simulink model "gmrt_002_muxdelay"  (Simulink 7.5) (continued)
== system gmrt_002_muxdelay == (continued)
      mask init:
      | pfb_fir_mux_init(gcb, ...
      |     'PFBSize', PFBSize, ...
      |     'mux_inputs', mux_inputs, ...
      |     'TotalTaps', TotalTaps, ...
      |     'WindowType', WindowType, ...
      |     'n_inputs', n_inputs, ...
      |     'MakeBiplex', MakeBiplex, ...
      |     'BitWidthIn', BitWidthIn, ...
      |     'BitWidthOut', BitWidthOut, ...
      |     'CoeffBitWidth', CoeffBitWidth, ...
      |     'CoeffDistMem', CoeffDistMem, ...
      |     'add_latency', add_latency, ...
      |     'mult_latency', mult_latency, ...
      |     'bram_latency', bram_latency, ...
      |     'quantization', quantization, ...
      |     'fwidth', fwidth,...
      |     'specify_mult', specify_mult,...
      |     'mult_spec', mult_spec);
      settings: AttributesFormatString=taps=4, add_latency=1
      mask Help: eval('xlWeb(''http://casper.berkeley.edu/wiki/Pfb_fir'')')
      mask StyleString: edit,edit,edit,popup(bartlett|barthannwin|blackman|blackmanharris|bohamwin|chebwin|flattopwin|gausswin|hamming|hann|kaiser|nuttallwin|parzenwin|rectwin|tukeywin|triang|userwindow),edit,edit,edit,edit,edit,edit,edit,edit,edit,popup(Truncate|Round  (unbiased: +/- Inf)|Round  (unbiased: Even Values)),edit,checkbox,edit
      mask CallbackString:
      | ||%num_taps = eval(get_param(gcb, 'TotalTaps'));
      | %old_vec = eval(get_param(gcb, 'mult_spec'));
      | 
      | %vec = 2.*ones(1,num_taps);
      | %old_size = length(old_vec);
      | 
      | %if(old_size <= num_taps),
      | %   new_size = old_size;
      | %else,
      | %    new_size = num_taps;
      | %end
      | 
      | %for n = 1:new_size, vec(n) = old_vec(n); end
      | %set_param(gcb, 'mult_spec', mat2str(vec));||||||||||||%num_taps = eval(get_param(gcb, 'TotalTaps'));
      | %old_vec = eval(get_param(gcb, 'mult_spec'));
      | 
      | %vec = 2.*ones(1,num_taps);
      | %old_size = length(old_vec);
      | 
      | %if(old_size <= num_taps),
      | %    new_size = old_size;
      | %else,
      | %    new_size = num_taps;
      | %end
      | 
      | %for n = 1:new_size, vec(n) = old_vec(n); end
      | %set_param(gcb, 'mult_spec', mat2str(vec));|%specify_mult = get_param(gcb,'specify_mult');
      | %if( strcmp(specify_mult,'off')),
      | %    set_param(gcb, 'MaskEnables', {'on','on','on','on','on','on','on','on','on','on','on','on','on','on','on','off'});
      | %else
      | %    set_param(gcb, 'MaskEnables', {'on','on','on','on','on','on','on','on','on','on','on','on','on','on','on','on'});
      | %end
      | 
      | |%specify_mult = get_param(gcb,'specify_mult');
      | %if( strcmp(specify_mult,'off')),
      | %    set_param(gcb, 'MaskEnables', {'on','on','on','on','on','on','on','on','on','on','on','on','on','on','on','off'});
      | %else
      | %    set_param(gcb, 'MaskEnables', {'on','on','on','on','on','on','on','on','on','on','on','on','on','on','on','on'});
      | %end
  pfb_fir_mux2 : Subsystem  AncestorBlock=casper_library_pfbs/pfb_fir_mux  mask(pfb_fir_mux){PFBSize=10, mux_inputs=2, TotalTaps=4, WindowType=hamming, n_inputs=0, MakeBiplex=1, BitWidthIn=12, BitWidthOut=18, CoeffBitWidth=18, CoeffDistMem=0, add_latency=1, mult_latency=2, bram_latency=3, quantization=Round  (unbiased: +/- Inf), fwidth=1, specify_mult=off, mult_spec=[2                                      2]}
      mask prompts: PFBSize "Size of PFB: (2^? pnts)"; mux_inputs "Signals per input (2^?)"; TotalTaps "Total Number of Taps:"; WindowType "Windowing Function:"; n_inputs "Number of Simultaneous Inputs: (2^?)"; MakeBiplex "Make Biplex"; BitWidthIn "Input Bitwidth:"; BitWidthOut "Output Bitwidth:"; CoeffBitWidth "Coefficient Bitwidth:"; CoeffDistMem "Use Distributed Memory for Coeffs"; add_latency "Add Latency"; mult_latency "Mult Latency"; bram_latency "BRAM Latency"; quantization "Quantization Behavior"; fwidth "Bin Width Scaling (normal=1)"; specify_mult "Specify multiplier use (behavioral if not specified)"; mult_spec "Multiplier specification (0=core, 1=embedded, 2=behavioural) (left=1st tap)"
      mask init: same as gmrt_002_muxdelay/pfb_fir_mux1
      settings: AttributesFormatString=taps=4, add_latency=1
      mask Help: eval('xlWeb(''http://casper.berkeley.edu/wiki/Pfb_fir'')')
      mask StyleString: edit,edit,edit,popup(bartlett|barthannwin|blackman|blackmanharris|bohamwin|chebwin|flattopwin|gausswin|hamming|hann|kaiser|nuttallwin|parzenwin|rectwin|tukeywin|triang|userwindow),edit,edit,edit,edit,edit,edit,edit,edit,edit,popup(Truncate|Round  (unbiased: +/- Inf)|Round  (unbiased: Even Values)),edit,checkbox,edit
      mask CallbackString:
      | ||%num_taps = eval(get_param(gcb, 'TotalTaps'));
      | %old_vec = eval(get_param(gcb, 'mult_spec'));
      | 
      | %vec = 2.*ones(1,num_taps);
      | %old_size = length(old_vec);
      | 
      | %if(old_size <= num_taps),
      | %   new_size = old_size;
      | %else,
      | %    new_size = num_taps;
      | %end
      | 
      | %for n = 1:new_size, vec(n) = old_vec(n); end
      | %set_param(gcb, 'mult_spec', mat2str(vec));||||||||||||%num_taps = eval(get_param(gcb, 'TotalTaps'));
      | %old_vec = eval(get_param(gcb, 'mult_spec'));
      | 
      | %vec = 2.*ones(1,num_taps);
      | %old_size = length(old_vec);
      | 
      | %if(old_size <= num_taps),
      | %    new_size = old_size;
      | %else,
      | %    new_size = num_taps;
      | %end
      | 
      | %for n = 1:new_size, vec(n) = old_vec(n); end
      | %set_param(gcb, 'mult_spec', mat2str(vec));|%specify_mult = get_param(gcb,'specify_mult');
      | %if( strcmp(specify_mult,'off')),
      | %    set_param(gcb, 'MaskEnables', {'on','on','on','on','on','on','on','on','on','on','on','on','on','on','on','off'});
      | %else
      | %    set_param(gcb, 'MaskEnables', {'on','on','on','on','on','on','on','on','on','on','on','on','on','on','on','on'});
      | %end
      | 
      | |%specify_mult = get_param(gcb,'specify_mult');
      | %if( strcmp(specify_mult,'off')),
      | %    set_param(gcb, 'MaskEnables', {'on','on','on','on','on','on','on','on','on','on','on','on','on','on','on','off'});
      | %else
      | %    set_param(gcb, 'MaskEnables', {'on','on','on','on','on','on','on','on','on','on','on','on','on','on','on','on'});
      | %end
  pfb_fir_mux3 : Subsystem  AncestorBlock=casper_library_pfbs/pfb_fir_mux  mask(pfb_fir_mux){PFBSize=10, mux_inputs=2, TotalTaps=4, WindowType=hamming, n_inputs=0, MakeBiplex=1, BitWidthIn=12, BitWidthOut=18, CoeffBitWidth=18, CoeffDistMem=0, add_latency=1, mult_latency=2, bram_latency=3, quantization=Round  (unbiased: +/- Inf), fwidth=1, specify_mult=off, mult_spec=[2                                      2]}
      mask prompts: PFBSize "Size of PFB: (2^? pnts)"; mux_inputs "Signals per input (2^?)"; TotalTaps "Total Number of Taps:"; WindowType "Windowing Function:"; n_inputs "Number of Simultaneous Inputs: (2^?)"; MakeBiplex "Make Biplex"; BitWidthIn "Input Bitwidth:"; BitWidthOut "Output Bitwidth:"; CoeffBitWidth "Coefficient Bitwidth:"; CoeffDistMem "Use Distributed Memory for Coeffs"; add_latency "Add Latency"; mult_latency "Mult Latency"; bram_latency "BRAM Latency"; quantization "Quantization Behavior"; fwidth "Bin Width Scaling (normal=1)"; specify_mult "Specify multiplier use (behavioral if not specified)"; mult_spec "Multiplier specification (0=core, 1=embedded, 2=behavioural) (left=1st tap)"
      mask init: same as gmrt_002_muxdelay/pfb_fir_mux1
      settings: AttributesFormatString=taps=4, add_latency=1
      mask Help: eval('xlWeb(''http://casper.berkeley.edu/wiki/Pfb_fir'')')
      mask StyleString: edit,edit,edit,popup(bartlett|barthannwin|blackman|blackmanharris|bohamwin|chebwin|flattopwin|gausswin|hamming|hann|kaiser|nuttallwin|parzenwin|rectwin|tukeywin|triang|userwindow),edit,edit,edit,edit,edit,edit,edit,edit,edit,popup(Truncate|Round  (unbiased: +/- Inf)|Round  (unbiased: Even Values)),edit,checkbox,edit
      mask CallbackString:
      | ||%num_taps = eval(get_param(gcb, 'TotalTaps'));
      | %old_vec = eval(get_param(gcb, 'mult_spec'));
      | 
      | %vec = 2.*ones(1,num_taps);
      | %old_size = length(old_vec);
      | 
      | %if(old_size <= num_taps),
      | %   new_size = old_size;
      | %else,
      | %    new_size = num_taps;
      | %end
      | 
      | %for n = 1:new_size, vec(n) = old_vec(n); end
      | %set_param(gcb, 'mult_spec', mat2str(vec));||||||||||||%num_taps = eval(get_param(gcb, 'TotalTaps'));
      | %old_vec = eval(get_param(gcb, 'mult_spec'));
      | 
      | %vec = 2.*ones(1,num_taps);
      | %old_size = length(old_vec);
      | 
      | %if(old_size <= num_taps),
      | %    new_size = old_size;
      | %else,
      | %    new_size = num_taps;
      | %end
      | 
      | %for n = 1:new_size, vec(n) = old_vec(n); end
      | %set_param(gcb, 'mult_spec', mat2str(vec));|%specify_mult = get_param(gcb,'specify_mult');
      | %if( strcmp(specify_mult,'off')),
      | %    set_param(gcb, 'MaskEnables', {'on','on','on','on','on','on','on','on','on','on','on','on','on','on','on','off'});
      | %else
      | %    set_param(gcb, 'MaskEnables', {'on','on','on','on','on','on','on','on','on','on','on','on','on','on','on','on'});
      | %end
      | 
      | |%specify_mult = get_param(gcb,'specify_mult');
      | %if( strcmp(specify_mult,'off')),
      | %    set_param(gcb, 'MaskEnables', {'on','on','on','on','on','on','on','on','on','on','on','on','on','on','on','off'});
      | %else
      | %    set_param(gcb, 'MaskEnables', {'on','on','on','on','on','on','on','on','on','on','on','on','on','on','on','on'});
      | %end
  pfb_fir_mux4 : Subsystem  AncestorBlock=casper_library_pfbs/pfb_fir_mux  mask(pfb_fir_mux){PFBSize=10, mux_inputs=2, TotalTaps=4, WindowType=hamming, n_inputs=0, MakeBiplex=1, BitWidthIn=12, BitWidthOut=18, CoeffBitWidth=18, CoeffDistMem=0, add_latency=1, mult_latency=2, bram_latency=3, quantization=Round  (unbiased: +/- Inf), fwidth=1, specify_mult=off, mult_spec=[2                                      2]}
      mask prompts: PFBSize "Size of PFB: (2^? pnts)"; mux_inputs "Signals per input (2^?)"; TotalTaps "Total Number of Taps:"; WindowType "Windowing Function:"; n_inputs "Number of Simultaneous Inputs: (2^?)"; MakeBiplex "Make Biplex"; BitWidthIn "Input Bitwidth:"; BitWidthOut "Output Bitwidth:"; CoeffBitWidth "Coefficient Bitwidth:"; CoeffDistMem "Use Distributed Memory for Coeffs"; add_latency "Add Latency"; mult_latency "Mult Latency"; bram_latency "BRAM Latency"; quantization "Quantization Behavior"; fwidth "Bin Width Scaling (normal=1)"; specify_mult "Specify multiplier use (behavioral if not specified)"; mult_spec "Multiplier specification (0=core, 1=embedded, 2=behavioural) (left=1st tap)"
      mask init: same as gmrt_002_muxdelay/pfb_fir_mux1
      settings: AttributesFormatString=taps=4, add_latency=1
      mask Help: eval('xlWeb(''http://casper.berkeley.edu/wiki/Pfb_fir'')')
      mask StyleString: edit,edit,edit,popup(bartlett|barthannwin|blackman|blackmanharris|bohamwin|chebwin|flattopwin|gausswin|hamming|hann|kaiser|nuttallwin|parzenwin|rectwin|tukeywin|triang|userwindow),edit,edit,edit,edit,edit,edit,edit,edit,edit,popup(Truncate|Round  (unbiased: +/- Inf)|Round  (unbiased: Even Values)),edit,checkbox,edit
      mask CallbackString:
      | ||%num_taps = eval(get_param(gcb, 'TotalTaps'));
      | %old_vec = eval(get_param(gcb, 'mult_spec'));
      | 
      | %vec = 2.*ones(1,num_taps);
      | %old_size = length(old_vec);
      | 
      | %if(old_size <= num_taps),
      | %   new_size = old_size;
      | %else,
      | %    new_size = num_taps;
      | %end
      | 
      | %for n = 1:new_size, vec(n) = old_vec(n); end
      | %set_param(gcb, 'mult_spec', mat2str(vec));||||||||||||%num_taps = eval(get_param(gcb, 'TotalTaps'));
      | %old_vec = eval(get_param(gcb, 'mult_spec'));
      | 
      | %vec = 2.*ones(1,num_taps);
      | %old_size = length(old_vec);
      | 
      | %if(old_size <= num_taps),
      | %    new_size = old_size;
      | %else,
      | %    new_size = num_taps;
      | %end
      | 
      | %for n = 1:new_size, vec(n) = old_vec(n); end
      | %set_param(gcb, 'mult_spec', mat2str(vec));|%specify_mult = get_param(gcb,'specify_mult');
      | %if( strcmp(specify_mult,'off')),
      | %    set_param(gcb, 'MaskEnables', {'on','on','on','on','on','on','on','on','on','on','on','on','on','on','on','off'});
      | %else
      | %    set_param(gcb, 'MaskEnables', {'on','on','on','on','on','on','on','on','on','on','on','on','on','on','on','on'});
      | %end
      | 
      | |%specify_mult = get_param(gcb,'specify_mult');
      | %if( strcmp(specify_mult,'off')),
      | %    set_param(gcb, 'MaskEnables', {'on','on','on','on','on','on','on','on','on','on','on','on','on','on','on','off'});
      | %else
      | %    set_param(gcb, 'MaskEnables', {'on','on','on','on','on','on','on','on','on','on','on','on','on','on','on','on'});
      | %end
  phase_EQ1 : Subsystem  mask{N=10+5 - 2, bram_lat=2, mult_lat=3, add_lat=2, chans_per_coeff=2}
      mask prompts: N "number of coefficients (2^?)"; bram_lat "bram latency"; mult_lat "multiplier latency"; add_lat "adder latency"; chans_per_coeff "channels per coeff (2^?)"
  pipeline : Subsystem  AncestorBlock=casper_library/Delays/pipeline  mask(pipeline){latency=1}
      mask help:
      | An explicitly laid-out delay line to avoid mapping to SRL16s
      | for use in pipelining to help achieve timing closure.
      mask init:
      | pipeline_init(gcb, 'latency', latency);
      mask Display:
      | port_label('input', 1, 'd');
      | port_label('output', 1, 'q');
      | disp(['Z^-', num2str(latency)]);
  pipeline1 : Subsystem  AncestorBlock=casper_library/Delays/pipeline  mask(pipeline){latency=2}
      mask help:
      | An explicitly laid-out delay line to avoid mapping to SRL16s
      | for use in pipelining to help achieve timing closure.
      mask init:
      | pipeline_init(gcb, 'latency', latency);
      mask Display:
      | port_label('input', 1, 'd');
      | port_label('output', 1, 'q');
      | disp(['Z^-', num2str(latency)]);
  pipeline10 : Subsystem  AncestorBlock=casper_library/Delays/pipeline  mask(pipeline){latency=1}
      mask help:
      | An explicitly laid-out delay line to avoid mapping to SRL16s
      | for use in pipelining to help achieve timing closure.
      mask init:
      | pipeline_init(gcb, 'latency', latency);
      mask Display:
      | port_label('input', 1, 'd');
      | port_label('output', 1, 'q');
      | disp(['Z^-', num2str(latency)]);
  pipeline11 : Subsystem  AncestorBlock=casper_library/Delays/pipeline  mask(pipeline){latency=1}
      mask help:
      | An explicitly laid-out delay line to avoid mapping to SRL16s
      | for use in pipelining to help achieve timing closure.
      mask init:
      | pipeline_init(gcb, 'latency', latency);
      mask Display:
      | port_label('input', 1, 'd');
      | port_label('output', 1, 'q');
      | disp(['Z^-', num2str(latency)]);
  pipeline12 : Subsystem  AncestorBlock=casper_library/Delays/pipeline  mask(pipeline){latency=1}
      mask help:
      | An explicitly laid-out delay line to avoid mapping to SRL16s
      | for use in pipelining to help achieve timing closure.
      mask init:
      | pipeline_init(gcb, 'latency', latency);
      mask Display:
      | port_label('input', 1, 'd');
      | port_label('output', 1, 'q');
      | disp(['Z^-', num2str(latency)]);
  pipeline13 : Subsystem  AncestorBlock=casper_library/Delays/pipeline  mask(pipeline){latency=1}
      mask help:
      | An explicitly laid-out delay line to avoid mapping to SRL16s
      | for use in pipelining to help achieve timing closure.
      mask init:
      | pipeline_init(gcb, 'latency', latency);
      mask Display:
      | port_label('input', 1, 'd');
      | port_label('output', 1, 'q');
      | disp(['Z^-', num2str(latency)]);
  pipeline14 : Subsystem  AncestorBlock=casper_library/Delays/pipeline  mask(pipeline){latency=1}
      mask help:
      | An explicitly laid-out delay line to avoid mapping to SRL16s
      | for use in pipelining to help achieve timing closure.
      mask init:
      | pipeline_init(gcb, 'latency', latency);
      mask Display:
      | port_label('input', 1, 'd');
      | port_label('output', 1, 'q');
      | disp(['Z^-', num2str(latency)]);
  pipeline15 : Subsystem  AncestorBlock=casper_library/Delays/pipeline  mask(pipeline){latency=1}
      mask help:
      | An explicitly laid-out delay line to avoid mapping to SRL16s
      | for use in pipelining to help achieve timing closure.
      mask init:
      | pipeline_init(gcb, 'latency', latency);
      mask Display:
      | port_label('input', 1, 'd');
      | port_label('output', 1, 'q');
      | disp(['Z^-', num2str(latency)]);
  pipeline16 : Subsystem  AncestorBlock=casper_library/Delays/pipeline  mask(pipeline){latency=1}
      mask help:
      | An explicitly laid-out delay line to avoid mapping to SRL16s
      | for use in pipelining to help achieve timing closure.
      mask init:
      | pipeline_init(gcb, 'latency', latency);
      mask Display:
      | port_label('input', 1, 'd');
      | port_label('output', 1, 'q');
      | disp(['Z^-', num2str(latency)]);
  pipeline17 : Subsystem  AncestorBlock=casper_library/Delays/pipeline  mask(pipeline){latency=2}
      mask help:
      | An explicitly laid-out delay line to avoid mapping to SRL16s
      | for use in pipelining to help achieve timing closure.
      mask init:
      | pipeline_init(gcb, 'latency', latency);
      mask Display:
      | port_label('input', 1, 'd');
      | port_label('output', 1, 'q');
      | disp(['Z^-', num2str(latency)]);
  pipeline4 : Subsystem  AncestorBlock=casper_library/Delays/pipeline  mask(pipeline){latency=1}
      mask help:
      | An explicitly laid-out delay line to avoid mapping to SRL16s
      | for use in pipelining to help achieve timing closure.
      mask init:
      | pipeline_init(gcb, 'latency', latency);
      mask Display:
      | port_label('input', 1, 'd');
      | port_label('output', 1, 'q');
      | disp(['Z^-', num2str(latency)]);
  pulse_ext : casper_library_misc/pulse_ext  SystemSampleTime=-1  pulse_len=2^26
  pulse_ext1 : casper_library_misc/pulse_ext  SystemSampleTime=-1  pulse_len=2^25
  q_snap : Subsystem
  sim_sync1 : ox_lib/sim_sync  SystemSampleTime=-1  delay=9
  sim_sync3 : ox_lib/sim_sync  SystemSampleTime=-1  delay=10
  sim_val : Subsystem  AncestorBlock=ox_lib/sim_sync  mask{val=2^11-1, bits=11}
      mask prompts: val "value"
  snap_ctrl : xps_library/software register  Tag=xps:sw_reg  SystemSampleTime=-1  io_dir=From Processor  arith_type=Unsigned  bitwidth=32  bin_pt=0  sample_period=1  gw_name=gmrt_001_intial_snap_ctrl_user_data_out
  sum_streams_x : Subsystem
  sum_streams_y : Subsystem
  sync_gen : Subsystem
  sync_gpio : xps_library/gpio  Tag=xps:gpio  SystemSampleTime=-1  io_group=ROACH:gpioa  io_dir=in  arith_type=Boolean  bitwidth=1  bin_pt=0  bit_index=6  sample_period=1  use_single_ended=off  use_ddr=off  reg_iob=on  reg_clk_phase=0  termination=Pulldown
  sync_gpio_io_sel : xps_library/gpio  Tag=xps:gpio  SystemSampleTime=-1  io_group=ROACH:gpioa_oe_n  io_dir=out  arith_type=Boolean  bitwidth=1  bin_pt=0  bit_index=0  sample_period=1  use_single_ended=off  use_ddr=off  reg_iob=on  reg_clk_phase=0  termination=Pulldown
  sync_led0 : xps_library/gpio  Tag=xps:gpio  SystemSampleTime=-1  io_group=ROACH:led  io_dir=out  arith_type=Boolean  bitwidth=1  bin_pt=0  bit_index=0  sample_period=1  use_single_ended=off  use_ddr=off  reg_iob=on  reg_clk_phase=0  termination=Pulldown
  sync_led1 : xps_library/gpio  Tag=xps:gpio  SystemSampleTime=-1  io_group=ROACH:led  io_dir=out  arith_type=Boolean  bitwidth=1  bin_pt=0  bit_index=1  sample_period=1  use_single_ended=off  use_ddr=off  reg_iob=on  reg_clk_phase=0  termination=Pulldown
  uncram : Subsystem  AncestorBlock=gavrt_library/uncram  mask(uncram){num_slice=8, slice_width=1, bin_pt=0, arith_type=0}
      mask prompts: num_slice "Number of slices"; slice_width "Slice Width"; bin_pt "Output Binary Point"; arith_type "Output Arithmetic Type (0=Unsigned, 1=Signed)"
      mask help: Takes a concatenated input and slices it up into even pieces and reinterprets them as signed fixed point numbers with a given binary point.
      mask init:
      | uncram_init(gcb, ...
      |     'num_slice', num_slice, ...
      |     'slice_width', slice_width, ...
      |     'bin_pt', bin_pt, ...
      |     'arith_type', arith_type);
      settings: AttributesFormatString=UFix_1_0
  x64_adc : Subsystem  Tag=xps:x64_adc  AncestorBlock=ox_lib/x64_adc  mask(x64_adc){adc_clk_rate=50, spi=off, ctrl_gpio=GPIO_B}
      mask prompts: adc_clk_rate "ADC clock rate (MHz)"; spi "Include SPI interface?"; ctrl_gpio "GPIO bank to use for control signals?"
      mask help: The ADC block converts analog inputs to digital outputs. Every clock cycle, the inputs are sampled and digitized to 8 bit binary point numbers in the range of [-1, 1) and are then output by the adc.
      mask init:
      | x64_adc_mask(gcb);
      mask Help: eval('xlWeb([getenv(''MLIB_ROOT''), ''\doc\html\sys_adc\sys_adc.html''])')
      mask StyleString: edit,checkbox,popup(GPIO_A|GPIO_B)
  wires:
  Constant20/1 -> ctrl_sw/1
  ctrl_sw/1 -> Slice/1, Slice4/1, Slice2/1, Slice12/1, Slice11/1, Slice5/1, Slice10/1, Slice13/1
  Slice4/1 -> pipeline15/1(d)
  adc_spi_ctrl/1 -> Slice3/1
  Slice3/1 -> x64_adc/18(adc_rst)
  x64_adc/17(chan_sync) -> Delay5/1, [adc_chan_sync_warn]
  [adc0_3i_in] -> adc_sw/1(in0)
  [adc0_3q_in] -> adc_sw/2(in1)
  [adc_chan_sync] -> Slice1/1
  Slice1/1 -> adc_sw/9(chan_sync)
  Slice/1 -> pipeline16/1(d)
  Slice2/1 -> pipeline14/1(d)
  sync_gen/1(sync) -> sim_sync3/1
  [arm_rst] -> sync_gen/2(arm)
  sync_gen/3(armed) -> [armed]
  Logical7/1 -> edge_detect/1
  sync_gpio/1 -> Logical7/1, edge_detect1/1
  [sync_rst] -> Logical7/2
  sim_sync3/1 -> adc_sync_align/1(sync)
  adc_sync_align/1(sync_out) -> [sync_gen], pulse_ext1/1
  sync_gen/2(mrst) -> sim_sync1/1
  adc_sync_align1/1(sync_out) -> [mrst]
  [adc_chan_sync] -> Slice7/1
  Concat5/1 -> pipeline/1(d)
  Slice12/1 -> pipeline13/1(d)
  Slice7/1 -> adc_sync_align/2(adc_sync), adc_sync_align1/2(adc_sync)
  adc_sim_sync/1(Out1) -> x64_adc/17(sim_sync)
  [adc_chan_sync] -> uncram/1(In)
  uncram/1(Out1) -> Logical1/1
  uncram/2(Out2) -> Logical1/2
  uncram/3(Out3) -> Logical1/3
  uncram/4(Out4) -> Logical1/4
  uncram/5(Out5) -> Logical1/5
  uncram/6(Out6) -> Logical1/6
  uncram/7(Out7) -> Logical1/7
  uncram/8(Out8) -> Logical1/8
  Logical1/1 -> Convert/1
  [sync_gen] -> Delay/1
  Delay/1 -> Logical2/1
  [adc_chan_sync] -> Slice9/1
  Slice9/1 -> Inverter/1
  Inverter/1 -> Logical2/2
  Logical2/1 -> Convert1/1
  Delay3/1 -> adc_sync_align1/1(sync)
  Concat6/1 -> adc_sync_test/1
  sim_sync1/1 -> Delay3/1
  Convert/1 -> ext21/1(in)
  Convert1/1 -> ext22/1(in)
  Slice11/1 -> pipeline12/1(d)
  x64_adc/1(dout0) -> Mux13/2, Mux13/3, Mux7/3, Mux6/3, Mux12/3, Mux11/3, Mux15/3, Mux14/3, Mux17/3, Mux16/3, Mux19/3, Mux18/3, Mux4/3, Mux5/3, Mux2/3, Mux3/3, Mux1/3
  x64_adc/2(dout1) -> Mux1/2
  x64_adc/3(dout2) -> Mux3/2
  x64_adc/4(dout3) -> Mux2/2
  x64_adc/5(dout4) -> Mux5/2
  x64_adc/6(dout5) -> Mux4/2
  x64_adc/7(dout6) -> Mux7/2
  x64_adc/8(dout7) -> Mux6/2
  [debug_sigs] -> Mux7/1, Mux6/1, Mux12/1, Mux11/1, Mux15/1, Mux14/1, Mux17/1, Mux16/1, Mux18/1, Mux19/1, Mux4/1, Mux5/1, Mux2/1, Mux3/1, Mux1/1, Mux13/1
  ext22/1(out) -> Concat6/2
  ext21/1(out) -> Concat6/1
  [adc4_7i_in] -> adc_sw/3(in2)
  [adc4_7q_in] -> adc_sw/4(in3)
  [adc8_11i_in] -> adc_sw/5(in4)
  [adc8_11q_in] -> adc_sw/6(in5)
  [adc12_15i_in] -> adc_sw/7(in6)
  [adc12_15q_in] -> adc_sw/8(in7)
  [fft_of_0] -> Logical/1
  [fft_of_1] -> Logical/2
  Logical/1 -> ext1/1(in)
  pipeline/1(q) -> of_reg/1
  adc_sw/1(adc_tv_out) -> [adc_tv]
  Conant/1 -> sync_gpio_io_sel/1
  edge_detect/1 -> sync_gen/1(rst)
  pipeline1/1(q) -> sync_led0/1
  edge_detect1/1 -> pulse_ext/1
  pulse_ext/1 -> pipeline1/1(d)
  pulse_ext1/1 -> pipeline17/1(d)
  phase_EQ1/1(sync_out) -> [phase_EQ_sync_out]
  fft_biplex_real_2x1/1(sync_out) -> [fft_sync_out]
  fft_biplex_real_2x2/4(of) -> [fft_of_1]
  fft_biplex_real_2x2/2(pol02_out) -> [fft_8_15x_out]
  fft_biplex_real_2x2/3(pol13_out) -> [fft_8_15y_out]
  [sb_sync_out] -> phase_EQ1/1(sync_in)
  [sb_high_h] -> phase_EQ1/2(dinA)
  [sb_low_h] -> phase_EQ1/3(dinB)
  [sb_high_v] -> phase_EQ1/4(dinC)
  [sb_low_v] -> phase_EQ1/5(dinD)
  phase_EQ1/2(doutA) -> [phase_EQ_h_i]
  phase_EQ1/3(doutB) -> [phase_EQ_h_q]
  phase_EQ1/4(doutC) -> [phase_EQ_v_i]
  phase_EQ1/5(doutD) -> [phase_EQ_v_q]
  [quant] -> i_snap/9(quant0)
  [fft_sync_out] -> i_snap/10(fft_sync)
  [amp_EQ_sync_out] -> i_snap/11(amp_sync)
  [phase_EQ_sync_out] -> i_snap/12(phase_sync)
  [quant_sync] -> i_snap/14(quant_sync)
  [fft_0_7h_i_out] -> i_snap/1(fft0)
  [fft_0_7v_i_out] -> i_snap/2(fft1)
  [amp_0_7h_i_out] -> i_snap/3(amp0)
  [amp_0_7v_i_out] -> i_snap/4(amp1)
  [phase_EQ_h_i] -> i_snap/5(phase0)
  [phase_EQ_v_i] -> i_snap/6(phase1)
  Logical3/1 -> ext2/1(in)
  [amp_of_0] -> Logical3/1
  [amp_of_1] -> Logical3/2
  [amp_of_2] -> Logical3/3
  [amp_of_3] -> Logical3/4
  [sb_of] -> ext3/1(in)
  c_to_ri/1 -> fft_biplex_real_2x2/3(pol0)
  c_to_ri/2 -> fft_biplex_real_2x2/4(pol1)
  c_to_ri1/1 -> fft_biplex_real_2x2/5(pol2)
  c_to_ri1/2 -> fft_biplex_real_2x2/6(pol3)
  [mux_sync_out] -> fft_biplex_real_2x2/1(sync)
  [shift] -> fft_biplex_real_2x2/2(shift)
  c_to_ri2/1 -> fft_biplex_real_2x1/3(pol0)
  c_to_ri2/2 -> fft_biplex_real_2x1/4(pol1)
  c_to_ri3/1 -> fft_biplex_real_2x1/5(pol2)
  c_to_ri3/2 -> fft_biplex_real_2x1/6(pol3)
  [mux_sync_out] -> fft_biplex_real_2x1/1(sync)
  [shift] -> fft_biplex_real_2x1/2(shift)
  fft_biplex_real_2x1/2(pol02_out) -> [fft_0_7x_out]
  fft_biplex_real_2x1/3(pol13_out) -> [fft_0_7y_out]
  fft_biplex_real_2x1/4(of) -> [fft_of_0]
  [sb_high_h] -> i_snap/7(high0)
  [sb_high_v] -> i_snap/8(high1)
  [sb_sync_out] -> i_snap/13(subband_sync)
  Constant13/1 -> snap_ctrl/1
  [fft_0_7h_q_out] -> q_snap/1(fft0)
  [fft_0_7v_q_out] -> q_snap/2(fft1)
  [amp_0_7h_q_out] -> q_snap/3(amp0)
  [amp_0_7v_q_out] -> q_snap/4(amp1)
  [phase_EQ_h_q] -> q_snap/5(phase0)
  [phase_EQ_v_q] -> q_snap/6(phase1)
  [sb_low_h] -> q_snap/7(high0)
  [sb_low_v] -> q_snap/8(high1)
  [transpose_out] -> q_snap/9(quant0)
  [fft_sync_out] -> q_snap/10(fft_sync)
  [amp_EQ_sync_out] -> q_snap/11(amp_sync)
  [phase_EQ_sync_out] -> q_snap/12(phase_sync)
  [sb_sync_out] -> q_snap/13(subband_sync)
  [transpose_sync_out] -> q_snap/14(quant_sync)
  snap_ctrl/1 -> i_snap/15(ctrl_reg), q_snap/15(ctrl_reg)
  Slice5/1 -> pipeline11/1(d)
  Slice10/1 -> pipeline4/1(d)
  Slice13/1 -> pipeline10/1(d)
  phase_EQ1/6(doutAof) -> [phase_of_0]
  phase_EQ1/7(doutBof) -> [phase_of_1]
  phase_EQ1/8(doutCof) -> [phase_of_2]
  phase_EQ1/9(doutDof) -> [phase_of_3]
  [phase_of_0] -> Logical4/1
  [phase_of_1] -> Logical4/2
  [phase_of_2] -> Logical4/3
  [phase_of_3] -> Logical4/4
  pipeline10/1(q) -> edge_detect2/1
  pipeline4/1(q) -> edge_detect3/1
  pipeline12/1(q) -> [debug_sigs]
  pipeline11/1(q) -> [subband_sel]
  pipeline13/1(q) -> [xaui_rcv_rst]
  pipeline14/1(q) -> [sync_rst]
  pipeline15/1(q) -> [arm_rst]
  pipeline16/1(q) -> sim_val/1(sync_in)
  pipeline17/1(q) -> sync_led1/1
  edge_detect2/1 -> [mcnt_rst]
  edge_detect3/1 -> [acc_ctr_rst]
  ext3/1(out) -> Concat5/6
  ext2/1(out) -> Concat5/5
  ext1/1(out) -> Concat5/4
  [fftt_of] -> ext4/1(in)
  ext4/1(out) -> Concat5/3
  Logical4/1 -> ext5/1(in)
  ext5/1(out) -> Concat5/2
  ext6/1(out) -> Concat5/1
  [x_s_clash] -> ext6/1(in)
  Delay5/1 -> [adc_chan_sync]
  Product/1 -> x64_adc/1(sim0)
  Counter Free-Running/1 -> Product/2
  Constant/1 -> Product/1
  sim_val/1(sync_out) -> [shift]
  x64_adc/10(dout9) -> Mux11/2
  x64_adc/11(dout10) -> Mux15/2
  x64_adc/12(dout11) -> Mux14/2
  x64_adc/13(dout12) -> Mux17/2
  x64_adc/14(dout13) -> Mux16/2
  x64_adc/15(dout14) -> Mux19/2
  x64_adc/16(dout15) -> Mux18/2
  Concat1/1 -> coarse_delay/2(din0)
  Concat2/1 -> coarse_delay/3(din1)
  Concat3/1 -> coarse_delay/4(din2)
  Concat4/1 -> coarse_delay/5(din3)
  Concat7/1 -> coarse_delay/6(din4)
  Concat8/1 -> coarse_delay/7(din5)
  Concat9/1 -> coarse_delay/8(din6)
  Concat10/1 -> coarse_delay/9(din7)
  Mux13/1 -> [adc0_3x_in]
  Mux1/1 -> [adc4_7x_in]
  Mux3/1 -> [adc8_11x_in]
  Mux2/1 -> [adc12_15x_in]
  Mux5/1 -> [adc16_19x_in]
  Mux4/1 -> [adc20_23x_in]
  Mux7/1 -> [adc24_27x_in]
  Mux6/1 -> [adc28_32x_in]
  Mux12/1 -> [adc0_3y_in]
  Mux11/1 -> [adc4_7y_in]
  Mux15/1 -> [adc8_11y_in]
  Mux14/1 -> [adc12_15y_in]
  Mux17/1 -> [adc16_19y_in]
  Mux16/1 -> [adc20_23y_in]
  Mux19/1 -> [adc24_27y_in]
  Mux18/1 -> [adc28_32y_in]
  [adc_chan_sync_warn] -> coarse_delay/1(sync)
  [adc0_3x_in] -> Concat1/1
  [adc0_3y_in] -> Concat1/2
  [adc8_11x_in] -> Concat3/1
  [adc8_11y_in] -> Concat3/2
  [adc12_15x_in] -> Concat4/1
  [adc12_15y_in] -> Concat4/2
  [adc16_19x_in] -> Concat7/1
  [adc16_19y_in] -> Concat7/2
  [adc20_23x_in] -> Concat8/1
  [adc20_23y_in] -> Concat8/2
  [adc28_32x_in] -> Concat10/1
  [adc28_32y_in] -> Concat10/2
  [adc24_27x_in] -> Concat9/1
  [adc24_27y_in] -> Concat9/2
  [adc4_7x_in] -> Concat2/1
  [adc4_7y_in] -> Concat2/2
  coarse_delay/1(dout0) -> [dly_0_3xy_out]
  coarse_delay/2(dout1) -> [dly_4_7xy_out]
  coarse_delay/3(dout2) -> [dly_8_11xy_out]
  coarse_delay/4(dout3) -> [dly_12_15xy_out]
  coarse_delay/5(dout4) -> [dly_16_19xy_out]
  coarse_delay/6(dout5) -> [dly_20_23xy_out]
  coarse_delay/7(dout6) -> [dly_24_27xy_out]
  coarse_delay/8(dout7) -> [dly_28_31xy_out]
  [sync_gen] -> pfb_fir_mux1/1(sync)
  [sync_gen] -> pfb_fir_mux2/1(sync)
  [sync_gen] -> pfb_fir_mux3/1(sync)
  [sync_gen] -> pfb_fir_mux4/1(sync)
  [dly_0_3xy_out] -> pfb_fir_mux1/2(pol1_in1)
  [dly_4_7xy_out] -> pfb_fir_mux1/3(pol2_in1)
  [dly_8_11xy_out] -> pfb_fir_mux2/2(pol1_in1)
  [dly_12_15xy_out] -> pfb_fir_mux2/3(pol2_in1)
  [dly_16_19xy_out] -> pfb_fir_mux3/2(pol1_in1)
  [dly_20_23xy_out] -> pfb_fir_mux3/3(pol2_in1)
  [dly_24_27xy_out] -> pfb_fir_mux4/2(pol1_in1)
  [dly_28_31xy_out] -> pfb_fir_mux4/3(pol2_in1)
  pfb_fir_mux1/2(pol1_out1) -> [fir0_3xy_out]
  pfb_fir_mux1/1(sync_out) -> [fir_sync_out]
  pfb_fir_mux1/3(pol2_out1) -> [fir4_7xy_out]
  pfb_fir_mux2/2(pol1_out1) -> [fir8_11xy_out]
  pfb_fir_mux2/3(pol2_out1) -> [fir12_15xy_out]
  pfb_fir_mux3/2(pol1_out1) -> [fir16_19xy_out]
  pfb_fir_mux3/3(pol2_out1) -> [fir20_23xy_out]
  pfb_fir_mux4/2(pol1_out1) -> [fir24_27xy_out]
  pfb_fir_mux4/3(pol2_out1) -> [fir28_31xy_out]
  [fir_sync_out] -> mux_32_streams2/1(sync)
  [fir0_3xy_out] -> mux_32_streams2/2(in0)
  [fir4_7xy_out] -> mux_32_streams2/3(in1)
  [fir8_11xy_out] -> mux_32_streams2/4(in2)
  [fir12_15xy_out] -> mux_32_streams2/5(in3)
  [fir16_19xy_out] -> mux_32_streams2/6(in4)
  [fir20_23xy_out] -> mux_32_streams2/7(in5)
  [fir24_27xy_out] -> mux_32_streams2/8(in6)
  [fir28_31xy_out] -> mux_32_streams2/9(in7)
  fft_biplex_real_2x3/1(sync_out) -> [fft_sync_out]
  fft_biplex_real_2x4/4(of) -> [fft_of_1]
  fft_biplex_real_2x4/2(pol02_out) -> [fft_24_31_out]
  fft_biplex_real_2x4/3(pol13_out) -> [fft_24_31y_out]
  c_to_ri4/1 -> fft_biplex_real_2x4/3(pol0)
  c_to_ri4/2 -> fft_biplex_real_2x4/4(pol1)
  c_to_ri5/1 -> fft_biplex_real_2x4/5(pol2)
  c_to_ri5/2 -> fft_biplex_real_2x4/6(pol3)
  [mux_sync_out] -> fft_biplex_real_2x4/1(sync)
  [shift] -> fft_biplex_real_2x4/2(shift)
  c_to_ri6/1 -> fft_biplex_real_2x3/3(pol0)
  c_to_ri6/2 -> fft_biplex_real_2x3/4(pol1)
  c_to_ri7/1 -> fft_biplex_real_2x3/5(pol2)
  c_to_ri7/2 -> fft_biplex_real_2x3/6(pol3)
  [mux_sync_out] -> fft_biplex_real_2x3/1(sync)
  [shift] -> fft_biplex_real_2x3/2(shift)
  fft_biplex_real_2x3/2(pol02_out) -> [fft_16_23x_out]
  fft_biplex_real_2x3/3(pol13_out) -> [fft_16_23y_out]
  fft_biplex_real_2x3/4(of) -> [fft_of_0]
  mux_32_streams2/1(sync_out) -> [mux_sync_out]
  mux_32_streams2/2(Out0) -> c_to_ri2/1
  mux_32_streams2/3(Out1) -> c_to_ri3/1
  mux_32_streams2/4(Out2) -> c_to_ri/1
  mux_32_streams2/5(Out3) -> c_to_ri1/1
  mux_32_streams2/6(Out4) -> c_to_ri6/1
  mux_32_streams2/7(Out5) -> c_to_ri7/1
  mux_32_streams2/8(Out6) -> c_to_ri4/1
  mux_32_streams2/9(Out7) -> c_to_ri5/1
  x64_adc/9(dout8) -> Mux12/2
  [sb_sync_out] -> phase_EQ1/6(5clk_warn_in)
